simulink model "BMSModel"  (R2024b)
settings: Solver=VariableStepAuto  StartTime=0.0  StopTime=+inf  RelTol=1e-3
values from neighbouring files (symbols this model uses):
  CellCurrentLimitThreshold_Fault = 50    [startInit.m]
  CellCurrentLimitThreshold_Warning = 20    [startInit.m]
  CellTemperatureLimitThreshold_Fault = 65    [startInit.m]
  CellTemperatureLimitThreshold_Warning = 50    [startInit.m]
  CellVoltageLimitHigh_Fault = 4.25    [startInit.m]
  CellVoltageLimitHigh_Warning = 4.21    [startInit.m]
  CellVoltageLimitLow_Fault = 2.65    [startInit.m]
  CellVoltageLimitLow_Warning = 2.70    (= 2.7)    [startInit.m]
  ChargerParRes = 10000    [startInit.m]
  ChargerRes = 0    [startInit.m]
  DisChargerRes = 250    [startInit.m]
  DisInverterRes = 250    [startInit.m]
  DrivetrainEnDelay = 0.1    [startInit.m]
  InverterParRes = 10000    [startInit.m]
  InverterRes = 0    [startInit.m]
  PreChargerRes = 1000    [startInit.m]
  PreInverterRes = 1000    [startInit.m]
  VbattMin = 1    [startInit.m]
  VbattThersholdChrg = 0.8    [startInit.m]
  VbattThresholdDis = 0.2    [startInit.m]
undefined symbols (not defined in the file or in its folder): ContactorCmd, DisChrgCmd, DrivetrainEn, PassiveBalancingRes, PreChrgCmd, sigbuilder_block
libraries: fl_lib x135, nesl_utility x91, deeplib x11, batteryecm_lib x10, ee_lib x10
(part 13 of 13)

== matlab function BMSModel/Electric Vehicul Model/BMS model/SOC_Estimation/MATLAB Function10 ==
  settings: updateMethod=DISCRETE
  data: voltage input; current input; SOC_est output; temperature input
function SOC_est  = estimateSOC_EKF(voltage, current, temperature)
% ESTIMATESOC_EKF Estimates State of Charge using Extended Kalman Filter
% Inputs:
%   voltage: Measured battery voltage (V)
%   current: Measured battery current (A, positive for discharge)
%   temperature: Measured battery temperature (°C)
% Outputs:
%   SOC_est: Estimated State of Charge (0 to 1)

% Persistent variables to store EKF state
persistent x_est P_est
if isempty(x_est)
    x_est = [0.5]; % Initial SOC estimate (50%)
    P_est = eye(1) * 0.01; % Initial covariance
end

% Battery model parameters
Q_nom = 2.5; % Nominal capacity (Ah)
R0 = 0.1; % Internal resistance (Ohm)
SOC_points = linspace(0, 1, 11); % SOC points from 0 to 1
OCV_table = [3.0, 3.2, 3.4, 3.5, 3.6, 3.7, 3.8, 3.9, 4.0, 4.1, 4.2]; % OCV vs SOC
T_ref = 25; % Reference temperature (°C)
alpha = 0.005; % Temperature coefficient for resistance
dt = 1; % Fixed time step (seconds)

% Process noise covariance
Q = 1e-6; % Process noise for SOC

% Measurement noise covariance
R = 1e-4; % Measurement noise for voltage

% State transition function (SOC update)
f = @(x, I) x - (dt / (Q_nom * 3600)) * I; % Coulomb counting

% Measurement function (nonlinear OCV model)
h = @(x, I, T) interp1(SOC_points, OCV_table, x, 'linear', 'extrap') ...
    - I * R0 * (1 + alpha * (T - T_ref));

% Jacobian of state transition (df/dx)
A = 1; % Linear SOC update, derivative is 1

% Jacobian of measurement function (dh/dx)
dOCV_dSOC = @(x) (interp1(SOC_points(2:end), OCV_table(2:end), x, 'linear', 'extrap') - ...
    interp1(SOC_points(1:end-1), OCV_table(1:end-1), x, 'linear', 'extrap')) / ...
    (SOC_points(2) - SOC_points(1));
H = dOCV_dSOC(x_est);

% EKF Prediction Step
x_pred = f(x_est, current); % Predicted state
P_pred = A * P_est * A' + Q; % Predicted covariance

% EKF Update Step
z = voltage; % Measurement
z_pred = h(x_pred, current, temperature); % Predicted measurement
y = z - z_pred; % Measurement residual
S = H * P_pred * H' + R; % Residual covariance
K = P_pred * H' / S; % Kalman gain
x_est = x_pred + K * y; % Updated state estimate
P_est = (eye(1) - K * H) * P_pred; % Updated covariance

% Ensure SOC stays within [0, 1]
x_est = max(0, min(1, x_est));

% Output estimated SOC
SOC_est = x_est;
end

== matlab function BMSModel/Electric Vehicul Model/BMS model/SOC_Estimation/MATLAB Function11 ==
  settings: updateMethod=DISCRETE
  data: voltage input; current input; SOC_est output; temperature input
function SOC_est  = estimateSOC_EKF(voltage, current, temperature)
% ESTIMATESOC_EKF Estimates State of Charge using Extended Kalman Filter
% Inputs:
%   voltage: Measured battery voltage (V)
%   current: Measured battery current (A, positive for discharge)
%   temperature: Measured battery temperature (°C)
% Outputs:
%   SOC_est: Estimated State of Charge (0 to 1)

% Persistent variables to store EKF state
persistent x_est P_est
if isempty(x_est)
    x_est = [0.5]; % Initial SOC estimate (50%)
    P_est = eye(1) * 0.01; % Initial covariance
end

% Battery model parameters
Q_nom = 2.5; % Nominal capacity (Ah)
R0 = 0.1; % Internal resistance (Ohm)
SOC_points = linspace(0, 1, 11); % SOC points from 0 to 1
OCV_table = [3.0, 3.2, 3.4, 3.5, 3.6, 3.7, 3.8, 3.9, 4.0, 4.1, 4.2]; % OCV vs SOC
T_ref = 25; % Reference temperature (°C)
alpha = 0.005; % Temperature coefficient for resistance
dt = 1; % Fixed time step (seconds)

% Process noise covariance
Q = 1e-6; % Process noise for SOC

% Measurement noise covariance
R = 1e-4; % Measurement noise for voltage

% State transition function (SOC update)
f = @(x, I) x - (dt / (Q_nom * 3600)) * I; % Coulomb counting

% Measurement function (nonlinear OCV model)
h = @(x, I, T) interp1(SOC_points, OCV_table, x, 'linear', 'extrap') ...
    - I * R0 * (1 + alpha * (T - T_ref));

% Jacobian of state transition (df/dx)
A = 1; % Linear SOC update, derivative is 1

% Jacobian of measurement function (dh/dx)
dOCV_dSOC = @(x) (interp1(SOC_points(2:end), OCV_table(2:end), x, 'linear', 'extrap') - ...
    interp1(SOC_points(1:end-1), OCV_table(1:end-1), x, 'linear', 'extrap')) / ...
    (SOC_points(2) - SOC_points(1));
H = dOCV_dSOC(x_est);

% EKF Prediction Step
x_pred = f(x_est, current); % Predicted state
P_pred = A * P_est * A' + Q; % Predicted covariance

% EKF Update Step
z = voltage; % Measurement
z_pred = h(x_pred, current, temperature); % Predicted measurement
y = z - z_pred; % Measurement residual
S = H * P_pred * H' + R; % Residual covariance
K = P_pred * H' / S; % Kalman gain
x_est = x_pred + K * y; % Updated state estimate
P_est = (eye(1) - K * H) * P_pred; % Updated covariance

% Ensure SOC stays within [0, 1]
x_est = max(0, min(1, x_est));

% Output estimated SOC
SOC_est = x_est;
end

== matlab function BMSModel/Electric Vehicul Model/BMS model/SOC_Estimation/MATLAB Function2 ==
  settings: updateMethod=DISCRETE
  data: voltage input; current input; SOC_est output; temperature input
function SOC_est  = estimateSOC_EKF(voltage, current, temperature)
% ESTIMATESOC_EKF Estimates State of Charge using Extended Kalman Filter
% Inputs:
%   voltage: Measured battery voltage (V)
%   current: Measured battery current (A, positive for discharge)
%   temperature: Measured battery temperature (°C)
% Outputs:
%   SOC_est: Estimated State of Charge (0 to 1)

% Persistent variables to store EKF state
persistent x_est P_est
if isempty(x_est)
    x_est = [0.5]; % Initial SOC estimate (50%)
    P_est = eye(1) * 0.01; % Initial covariance
end

% Battery model parameters
Q_nom = 2.5; % Nominal capacity (Ah)
R0 = 0.1; % Internal resistance (Ohm)
SOC_points = linspace(0, 1, 11); % SOC points from 0 to 1
OCV_table = [3.0, 3.2, 3.4, 3.5, 3.6, 3.7, 3.8, 3.9, 4.0, 4.1, 4.2]; % OCV vs SOC
T_ref = 25; % Reference temperature (°C)
alpha = 0.005; % Temperature coefficient for resistance
dt = 1; % Fixed time step (seconds)

% Process noise covariance
Q = 1e-6; % Process noise for SOC

% Measurement noise covariance
R = 1e-4; % Measurement noise for voltage

% State transition function (SOC update)
f = @(x, I) x - (dt / (Q_nom * 3600)) * I; % Coulomb counting

% Measurement function (nonlinear OCV model)
h = @(x, I, T) interp1(SOC_points, OCV_table, x, 'linear', 'extrap') ...
    - I * R0 * (1 + alpha * (T - T_ref));

% Jacobian of state transition (df/dx)
A = 1; % Linear SOC update, derivative is 1

% Jacobian of measurement function (dh/dx)
dOCV_dSOC = @(x) (interp1(SOC_points(2:end), OCV_table(2:end), x, 'linear', 'extrap') - ...
    interp1(SOC_points(1:end-1), OCV_table(1:end-1), x, 'linear', 'extrap')) / ...
    (SOC_points(2) - SOC_points(1));
H = dOCV_dSOC(x_est);

% EKF Prediction Step
x_pred = f(x_est, current); % Predicted state
P_pred = A * P_est * A' + Q; % Predicted covariance

% EKF Update Step
z = voltage; % Measurement
z_pred = h(x_pred, current, temperature); % Predicted measurement
y = z - z_pred; % Measurement residual
S = H * P_pred * H' + R; % Residual covariance
K = P_pred * H' / S; % Kalman gain
x_est = x_pred + K * y; % Updated state estimate
P_est = (eye(1) - K * H) * P_pred; % Updated covariance

% Ensure SOC stays within [0, 1]
x_est = max(0, min(1, x_est));

% Output estimated SOC
SOC_est = x_est;
end

== matlab function BMSModel/Electric Vehicul Model/BMS model/SOC_Estimation/MATLAB Function3 ==
  settings: updateMethod=DISCRETE
  data: voltage input; current input; SOC_est output; temperature input
function SOC_est  = estimateSOC_EKF(voltage, current, temperature)
% ESTIMATESOC_EKF Estimates State of Charge using Extended Kalman Filter
% Inputs:
%   voltage: Measured battery voltage (V)
%   current: Measured battery current (A, positive for discharge)
%   temperature: Measured battery temperature (°C)
% Outputs:
%   SOC_est: Estimated State of Charge (0 to 1)

% Persistent variables to store EKF state
persistent x_est P_est
if isempty(x_est)
    x_est = [0.5]; % Initial SOC estimate (50%)
    P_est = eye(1) * 0.01; % Initial covariance
end

% Battery model parameters
Q_nom = 2.5; % Nominal capacity (Ah)
R0 = 0.1; % Internal resistance (Ohm)
SOC_points = linspace(0, 1, 11); % SOC points from 0 to 1
OCV_table = [3.0, 3.2, 3.4, 3.5, 3.6, 3.7, 3.8, 3.9, 4.0, 4.1, 4.2]; % OCV vs SOC
T_ref = 25; % Reference temperature (°C)
alpha = 0.005; % Temperature coefficient for resistance
dt = 1; % Fixed time step (seconds)

% Process noise covariance
Q = 1e-6; % Process noise for SOC

% Measurement noise covariance
R = 1e-4; % Measurement noise for voltage

% State transition function (SOC update)
f = @(x, I) x - (dt / (Q_nom * 3600)) * I; % Coulomb counting

% Measurement function (nonlinear OCV model)
h = @(x, I, T) interp1(SOC_points, OCV_table, x, 'linear', 'extrap') ...
    - I * R0 * (1 + alpha * (T - T_ref));

% Jacobian of state transition (df/dx)
A = 1; % Linear SOC update, derivative is 1

% Jacobian of measurement function (dh/dx)
dOCV_dSOC = @(x) (interp1(SOC_points(2:end), OCV_table(2:end), x, 'linear', 'extrap') - ...
    interp1(SOC_points(1:end-1), OCV_table(1:end-1), x, 'linear', 'extrap')) / ...
    (SOC_points(2) - SOC_points(1));
H = dOCV_dSOC(x_est);

% EKF Prediction Step
x_pred = f(x_est, current); % Predicted state
P_pred = A * P_est * A' + Q; % Predicted covariance

% EKF Update Step
z = voltage; % Measurement
z_pred = h(x_pred, current, temperature); % Predicted measurement
y = z - z_pred; % Measurement residual
S = H * P_pred * H' + R; % Residual covariance
K = P_pred * H' / S; % Kalman gain
x_est = x_pred + K * y; % Updated state estimate
P_est = (eye(1) - K * H) * P_pred; % Updated covariance

% Ensure SOC stays within [0, 1]
x_est = max(0, min(1, x_est));

% Output estimated SOC
SOC_est = x_est;
end

== matlab function BMSModel/Electric Vehicul Model/BMS model/SOC_Estimation/MATLAB Function4 ==
  settings: updateMethod=DISCRETE
  data: voltage input; current input; SOC_est output; temperature input
function SOC_est  = estimateSOC_EKF(voltage, current, temperature)
% ESTIMATESOC_EKF Estimates State of Charge using Extended Kalman Filter
% Inputs:
%   voltage: Measured battery voltage (V)
%   current: Measured battery current (A, positive for discharge)
%   temperature: Measured battery temperature (°C)
% Outputs:
%   SOC_est: Estimated State of Charge (0 to 1)

% Persistent variables to store EKF state
persistent x_est P_est
if isempty(x_est)
    x_est = [0.5]; % Initial SOC estimate (50%)
    P_est = eye(1) * 0.01; % Initial covariance
end

% Battery model parameters
Q_nom = 2.5; % Nominal capacity (Ah)
R0 = 0.1; % Internal resistance (Ohm)
SOC_points = linspace(0, 1, 11); % SOC points from 0 to 1
OCV_table = [3.0, 3.2, 3.4, 3.5, 3.6, 3.7, 3.8, 3.9, 4.0, 4.1, 4.2]; % OCV vs SOC
T_ref = 25; % Reference temperature (°C)
alpha = 0.005; % Temperature coefficient for resistance
dt = 1; % Fixed time step (seconds)

% Process noise covariance
Q = 1e-6; % Process noise for SOC

% Measurement noise covariance
R = 1e-4; % Measurement noise for voltage

% State transition function (SOC update)
f = @(x, I) x - (dt / (Q_nom * 3600)) * I; % Coulomb counting

% Measurement function (nonlinear OCV model)
h = @(x, I, T) interp1(SOC_points, OCV_table, x, 'linear', 'extrap') ...
    - I * R0 * (1 + alpha * (T - T_ref));

% Jacobian of state transition (df/dx)
A = 1; % Linear SOC update, derivative is 1

% Jacobian of measurement function (dh/dx)
dOCV_dSOC = @(x) (interp1(SOC_points(2:end), OCV_table(2:end), x, 'linear', 'extrap') - ...
    interp1(SOC_points(1:end-1), OCV_table(1:end-1), x, 'linear', 'extrap')) / ...
    (SOC_points(2) - SOC_points(1));
H = dOCV_dSOC(x_est);

% EKF Prediction Step
x_pred = f(x_est, current); % Predicted state
P_pred = A * P_est * A' + Q; % Predicted covariance

% EKF Update Step
z = voltage; % Measurement
z_pred = h(x_pred, current, temperature); % Predicted measurement
y = z - z_pred; % Measurement residual
S = H * P_pred * H' + R; % Residual covariance
K = P_pred * H' / S; % Kalman gain
x_est = x_pred + K * y; % Updated state estimate
P_est = (eye(1) - K * H) * P_pred; % Updated covariance

% Ensure SOC stays within [0, 1]
x_est = max(0, min(1, x_est));

% Output estimated SOC
SOC_est = x_est;
end

== matlab function BMSModel/Electric Vehicul Model/BMS model/SOC_Estimation/MATLAB Function5 ==
  settings: updateMethod=DISCRETE
  data: voltage input; current input; SOC_est output; temperature input
function SOC_est  = estimateSOC_EKF(voltage, current, temperature)
% ESTIMATESOC_EKF Estimates State of Charge using Extended Kalman Filter
% Inputs:
%   voltage: Measured battery voltage (V)
%   current: Measured battery current (A, positive for discharge)
%   temperature: Measured battery temperature (°C)
% Outputs:
%   SOC_est: Estimated State of Charge (0 to 1)

% Persistent variables to store EKF state
persistent x_est P_est
if isempty(x_est)
    x_est = [0.5]; % Initial SOC estimate (50%)
    P_est = eye(1) * 0.01; % Initial covariance
end

% Battery model parameters
Q_nom = 2.5; % Nominal capacity (Ah)
R0 = 0.1; % Internal resistance (Ohm)
SOC_points = linspace(0, 1, 11); % SOC points from 0 to 1
OCV_table = [3.0, 3.2, 3.4, 3.5, 3.6, 3.7, 3.8, 3.9, 4.0, 4.1, 4.2]; % OCV vs SOC
T_ref = 25; % Reference temperature (°C)
alpha = 0.005; % Temperature coefficient for resistance
dt = 1; % Fixed time step (seconds)

% Process noise covariance
Q = 1e-6; % Process noise for SOC

% Measurement noise covariance
R = 1e-4; % Measurement noise for voltage

% State transition function (SOC update)
f = @(x, I) x - (dt / (Q_nom * 3600)) * I; % Coulomb counting

% Measurement function (nonlinear OCV model)
h = @(x, I, T) interp1(SOC_points, OCV_table, x, 'linear', 'extrap') ...
    - I * R0 * (1 + alpha * (T - T_ref));

% Jacobian of state transition (df/dx)
A = 1; % Linear SOC update, derivative is 1

% Jacobian of measurement function (dh/dx)
dOCV_dSOC = @(x) (interp1(SOC_points(2:end), OCV_table(2:end), x, 'linear', 'extrap') - ...
    interp1(SOC_points(1:end-1), OCV_table(1:end-1), x, 'linear', 'extrap')) / ...
    (SOC_points(2) - SOC_points(1));
H = dOCV_dSOC(x_est);

% EKF Prediction Step
x_pred = f(x_est, current); % Predicted state
P_pred = A * P_est * A' + Q; % Predicted covariance

% EKF Update Step
z = voltage; % Measurement
z_pred = h(x_pred, current, temperature); % Predicted measurement
y = z - z_pred; % Measurement residual
S = H * P_pred * H' + R; % Residual covariance
K = P_pred * H' / S; % Kalman gain
x_est = x_pred + K * y; % Updated state estimate
P_est = (eye(1) - K * H) * P_pred; % Updated covariance

% Ensure SOC stays within [0, 1]
x_est = max(0, min(1, x_est));

% Output estimated SOC
SOC_est = x_est;
end

== matlab function BMSModel/Electric Vehicul Model/BMS model/SOC_Estimation/MATLAB Function6 ==
  settings: updateMethod=DISCRETE
  data: voltage input; current input; SOC_est output; temperature input
function SOC_est  = estimateSOC_EKF(voltage, current, temperature)
% ESTIMATESOC_EKF Estimates State of Charge using Extended Kalman Filter
% Inputs:
%   voltage: Measured battery voltage (V)
%   current: Measured battery current (A, positive for discharge)
%   temperature: Measured battery temperature (°C)
% Outputs:
%   SOC_est: Estimated State of Charge (0 to 1)

% Persistent variables to store EKF state
persistent x_est P_est
if isempty(x_est)
    x_est = [0.5]; % Initial SOC estimate (50%)
    P_est = eye(1) * 0.01; % Initial covariance
end

% Battery model parameters
Q_nom = 2.5; % Nominal capacity (Ah)
R0 = 0.1; % Internal resistance (Ohm)
SOC_points = linspace(0, 1, 11); % SOC points from 0 to 1
OCV_table = [3.0, 3.2, 3.4, 3.5, 3.6, 3.7, 3.8, 3.9, 4.0, 4.1, 4.2]; % OCV vs SOC
T_ref = 25; % Reference temperature (°C)
alpha = 0.005; % Temperature coefficient for resistance
dt = 1; % Fixed time step (seconds)

% Process noise covariance
Q = 1e-6; % Process noise for SOC

% Measurement noise covariance
R = 1e-4; % Measurement noise for voltage

% State transition function (SOC update)
f = @(x, I) x - (dt / (Q_nom * 3600)) * I; % Coulomb counting

% Measurement function (nonlinear OCV model)
h = @(x, I, T) interp1(SOC_points, OCV_table, x, 'linear', 'extrap') ...
    - I * R0 * (1 + alpha * (T - T_ref));

% Jacobian of state transition (df/dx)
A = 1; % Linear SOC update, derivative is 1

% Jacobian of measurement function (dh/dx)
dOCV_dSOC = @(x) (interp1(SOC_points(2:end), OCV_table(2:end), x, 'linear', 'extrap') - ...
    interp1(SOC_points(1:end-1), OCV_table(1:end-1), x, 'linear', 'extrap')) / ...
    (SOC_points(2) - SOC_points(1));
H = dOCV_dSOC(x_est);

% EKF Prediction Step
x_pred = f(x_est, current); % Predicted state
P_pred = A * P_est * A' + Q; % Predicted covariance

% EKF Update Step
z = voltage; % Measurement
z_pred = h(x_pred, current, temperature); % Predicted measurement
y = z - z_pred; % Measurement residual
S = H * P_pred * H' + R; % Residual covariance
K = P_pred * H' / S; % Kalman gain
x_est = x_pred + K * y; % Updated state estimate
P_est = (eye(1) - K * H) * P_pred; % Updated covariance

% Ensure SOC stays within [0, 1]
x_est = max(0, min(1, x_est));

% Output estimated SOC
SOC_est = x_est;
end

== matlab function BMSModel/Electric Vehicul Model/BMS model/SOC_Estimation/MATLAB Function7 ==
  settings: updateMethod=DISCRETE
  data: voltage input; current input; SOC_est output; temperature input
function SOC_est  = estimateSOC_EKF(voltage, current, temperature)
% ESTIMATESOC_EKF Estimates State of Charge using Extended Kalman Filter
% Inputs:
%   voltage: Measured battery voltage (V)
%   current: Measured battery current (A, positive for discharge)
%   temperature: Measured battery temperature (°C)
% Outputs:
%   SOC_est: Estimated State of Charge (0 to 1)

% Persistent variables to store EKF state
persistent x_est P_est
if isempty(x_est)
    x_est = [0.5]; % Initial SOC estimate (50%)
    P_est = eye(1) * 0.01; % Initial covariance
end

% Battery model parameters
Q_nom = 2.5; % Nominal capacity (Ah)
R0 = 0.1; % Internal resistance (Ohm)
SOC_points = linspace(0, 1, 11); % SOC points from 0 to 1
OCV_table = [3.0, 3.2, 3.4, 3.5, 3.6, 3.7, 3.8, 3.9, 4.0, 4.1, 4.2]; % OCV vs SOC
T_ref = 25; % Reference temperature (°C)
alpha = 0.005; % Temperature coefficient for resistance
dt = 1; % Fixed time step (seconds)

% Process noise covariance
Q = 1e-6; % Process noise for SOC

% Measurement noise covariance
R = 1e-4; % Measurement noise for voltage

% State transition function (SOC update)
f = @(x, I) x - (dt / (Q_nom * 3600)) * I; % Coulomb counting

% Measurement function (nonlinear OCV model)
h = @(x, I, T) interp1(SOC_points, OCV_table, x, 'linear', 'extrap') ...
    - I * R0 * (1 + alpha * (T - T_ref));

% Jacobian of state transition (df/dx)
A = 1; % Linear SOC update, derivative is 1

% Jacobian of measurement function (dh/dx)
dOCV_dSOC = @(x) (interp1(SOC_points(2:end), OCV_table(2:end), x, 'linear', 'extrap') - ...
    interp1(SOC_points(1:end-1), OCV_table(1:end-1), x, 'linear', 'extrap')) / ...
    (SOC_points(2) - SOC_points(1));
H = dOCV_dSOC(x_est);

% EKF Prediction Step
x_pred = f(x_est, current); % Predicted state
P_pred = A * P_est * A' + Q; % Predicted covariance

% EKF Update Step
z = voltage; % Measurement
z_pred = h(x_pred, current, temperature); % Predicted measurement
y = z - z_pred; % Measurement residual
S = H * P_pred * H' + R; % Residual covariance
K = P_pred * H' / S; % Kalman gain
x_est = x_pred + K * y; % Updated state estimate
P_est = (eye(1) - K * H) * P_pred; % Updated covariance

% Ensure SOC stays within [0, 1]
x_est = max(0, min(1, x_est));

% Output estimated SOC
SOC_est = x_est;
end

== matlab function BMSModel/Electric Vehicul Model/BMS model/SOC_Estimation/MATLAB Function8 ==
  settings: updateMethod=DISCRETE
  data: voltage input; current input; SOC_est output; temperature input
function SOC_est  = estimateSOC_EKF(voltage, current, temperature)
% ESTIMATESOC_EKF Estimates State of Charge using Extended Kalman Filter
% Inputs:
%   voltage: Measured battery voltage (V)
%   current: Measured battery current (A, positive for discharge)
%   temperature: Measured battery temperature (°C)
% Outputs:
%   SOC_est: Estimated State of Charge (0 to 1)

% Persistent variables to store EKF state
persistent x_est P_est
if isempty(x_est)
    x_est = [0.5]; % Initial SOC estimate (50%)
    P_est = eye(1) * 0.01; % Initial covariance
end

% Battery model parameters
Q_nom = 2.5; % Nominal capacity (Ah)
R0 = 0.1; % Internal resistance (Ohm)
SOC_points = linspace(0, 1, 11); % SOC points from 0 to 1
OCV_table = [3.0, 3.2, 3.4, 3.5, 3.6, 3.7, 3.8, 3.9, 4.0, 4.1, 4.2]; % OCV vs SOC
T_ref = 25; % Reference temperature (°C)
alpha = 0.005; % Temperature coefficient for resistance
dt = 1; % Fixed time step (seconds)

% Process noise covariance
Q = 1e-6; % Process noise for SOC

% Measurement noise covariance
R = 1e-4; % Measurement noise for voltage

% State transition function (SOC update)
f = @(x, I) x - (dt / (Q_nom * 3600)) * I; % Coulomb counting

% Measurement function (nonlinear OCV model)
h = @(x, I, T) interp1(SOC_points, OCV_table, x, 'linear', 'extrap') ...
    - I * R0 * (1 + alpha * (T - T_ref));

% Jacobian of state transition (df/dx)
A = 1; % Linear SOC update, derivative is 1

% Jacobian of measurement function (dh/dx)
dOCV_dSOC = @(x) (interp1(SOC_points(2:end), OCV_table(2:end), x, 'linear', 'extrap') - ...
    interp1(SOC_points(1:end-1), OCV_table(1:end-1), x, 'linear', 'extrap')) / ...
    (SOC_points(2) - SOC_points(1));
H = dOCV_dSOC(x_est);

% EKF Prediction Step
x_pred = f(x_est, current); % Predicted state
P_pred = A * P_est * A' + Q; % Predicted covariance

% EKF Update Step
z = voltage; % Measurement
z_pred = h(x_pred, current, temperature); % Predicted measurement
y = z - z_pred; % Measurement residual
S = H * P_pred * H' + R; % Residual covariance
K = P_pred * H' / S; % Kalman gain
x_est = x_pred + K * y; % Updated state estimate
P_est = (eye(1) - K * H) * P_pred; % Updated covariance

% Ensure SOC stays within [0, 1]
x_est = max(0, min(1, x_est));

% Output estimated SOC
SOC_est = x_est;
end

== matlab function BMSModel/Electric Vehicul Model/BMS model/SOC_Estimation/MATLAB Function9 ==
  settings: updateMethod=DISCRETE
  data: voltage input; current input; SOC_est output; temperature input
function SOC_est  = estimateSOC_EKF(voltage, current, temperature)
% ESTIMATESOC_EKF Estimates State of Charge using Extended Kalman Filter
% Inputs:
%   voltage: Measured battery voltage (V)
%   current: Measured battery current (A, positive for discharge)
%   temperature: Measured battery temperature (°C)
% Outputs:
%   SOC_est: Estimated State of Charge (0 to 1)

% Persistent variables to store EKF state
persistent x_est P_est
if isempty(x_est)
    x_est = [0.5]; % Initial SOC estimate (50%)
    P_est = eye(1) * 0.01; % Initial covariance
end

% Battery model parameters
Q_nom = 2.5; % Nominal capacity (Ah)
R0 = 0.1; % Internal resistance (Ohm)
SOC_points = linspace(0, 1, 11); % SOC points from 0 to 1
OCV_table = [3.0, 3.2, 3.4, 3.5, 3.6, 3.7, 3.8, 3.9, 4.0, 4.1, 4.2]; % OCV vs SOC
T_ref = 25; % Reference temperature (°C)
alpha = 0.005; % Temperature coefficient for resistance
dt = 1; % Fixed time step (seconds)

% Process noise covariance
Q = 1e-6; % Process noise for SOC

% Measurement noise covariance
R = 1e-4; % Measurement noise for voltage

% State transition function (SOC update)
f = @(x, I) x - (dt / (Q_nom * 3600)) * I; % Coulomb counting

% Measurement function (nonlinear OCV model)
h = @(x, I, T) interp1(SOC_points, OCV_table, x, 'linear', 'extrap') ...
    - I * R0 * (1 + alpha * (T - T_ref));

% Jacobian of state transition (df/dx)
A = 1; % Linear SOC update, derivative is 1

% Jacobian of measurement function (dh/dx)
dOCV_dSOC = @(x) (interp1(SOC_points(2:end), OCV_table(2:end), x, 'linear', 'extrap') - ...
    interp1(SOC_points(1:end-1), OCV_table(1:end-1), x, 'linear', 'extrap')) / ...
    (SOC_points(2) - SOC_points(1));
H = dOCV_dSOC(x_est);

% EKF Prediction Step
x_pred = f(x_est, current); % Predicted state
P_pred = A * P_est * A' + Q; % Predicted covariance

% EKF Update Step
z = voltage; % Measurement
z_pred = h(x_pred, current, temperature); % Predicted measurement
y = z - z_pred; % Measurement residual
S = H * P_pred * H' + R; % Residual covariance
K = P_pred * H' / S; % Kalman gain
x_est = x_pred + K * y; % Updated state estimate
P_est = (eye(1) - K * H) * P_pred; % Updated covariance

% Ensure SOC stays within [0, 1]
x_est = max(0, min(1, x_est));

% Output estimated SOC
SOC_est = x_est;
end

== matlab function BMSModel/Test Senarois/MATLAB Function ==
  data: faultType input; SCInjVector output; ModelNumber input; cellNumber input; OCInjVector output
function [SCInjVector, OCInjVector] = faultInjection(faultType, ModelNumber, cellNumber)

switch(faultType)
    case 0
        SCInjVector=false(1,10);
        OCInjVector=false(1,10);
    case 1
        SCInjVector=false(1,10);
        OCInjVector=false(1,10);
        SCInjVector(ModelNumber, cellNumber)=true;
    case 2
        SCInjVector=false(1,10);
        OCInjVector=false(1,10);
        OCInjVector(ModelNumber, cellNumber)=true;
    otherwise 
        SCInjVector=false(1,10);
        OCInjVector=false(1,10);
end

== matlab function BMSModel/Electric Vehicul Model/BMS model/Faults detection/MATLAB Function ==
  data: minInput input; W output; F output; CellVoltageLimitLow_Warning parameter; CellVoltageLimitLow_Fault parameter
function [W,F] = underVoltage(minInput,CellVoltageLimitLow_Warning, CellVoltageLimitLow_Fault)
W=0;
F=0;
if minInput <= CellVoltageLimitLow_Warning && minInput > CellVoltageLimitLow_Fault
    W=1;
    F=0;
elseif minInput <= CellVoltageLimitLow_Fault
    W=0;
    F=1;
end
end

== matlab function BMSModel/Electric Vehicul Model/BMS model/Faults detection/MATLAB Function1 ==
  data: maxInput input; W output; F output; CellVoltageLimitHigh_Warning parameter; CellVoltageLimitHigh_Fault parameter
function [W, F] = overVoltage( maxInput,CellVoltageLimitHigh_Warning, CellVoltageLimitHigh_Fault)
W=0;
F=0;
if maxInput >= CellVoltageLimitHigh_Warning && maxInput < CellVoltageLimitHigh_Fault

    W=1;
    F=0;
elseif maxInput >= CellVoltageLimitHigh_Fault
    W=0;
    F=1;
end

end
